simulink library "lib_all"  (Simulink 7.6)
settings: Solver=ode45  SolverMode=SingleTasking  StartTime=0.0  StopTime=10.0  RelTol=1e-3
values from neighbouring files (symbols this model uses):
  map_lib_03 = [6.8679 7.0159 7.4571 8.1849 9.1892 10.456 11.97 13.71 15.655 17.781 20.063 22.474 ... (803 elements, 73x11)]    [scr_simdata.mat]    (also in scr_simdata_all.mat)
  map_lib_06 = [2.5784 2.6727 2.9588 3.4453 4.1444 5.068 6.226 7.6242 9.2634 11.139 13.24 15.552 ... (803 elements, 73x11)]    [scr_simdata.mat]    (also in scr_simdata_all.mat)
  map_lib_09 = [0.0025784 0.0026727 0.0029588 0.0034453 0.0041444 0.005068 0.006226 0.0076242 0.0092634 0.011139 0.01324 0.015552 ... (803 elements, 73x11)]    [scr_simdata.mat]    (also in scr_simdata_all.mat)
  map_lib_10 = [2.74 2.75 2.76 2.77 2.78 2.79 2.8 2.81 2.82 2.83 2.84 2.85 ... (81 elements, 1x81)]    [scr_simdata.mat]    (also in scr_simdata_all.mat)
  map_lib_11 = [1.1983 1.2027 1.207 1.2113 1.2156 1.2198 1.2241 1.2283 1.2326 1.2368 1.241 1.2451 ... (81 elements, 1x81)]    [scr_simdata.mat]    (also in scr_simdata_all.mat)
  map_lib_12 = [2.74 2.75 2.76 2.77 2.78 2.79 2.8 2.81 2.82 2.83 2.84 2.85 ... (81 elements, 1x81)]    [scr_simdata.mat]    (also in scr_simdata_all.mat)
  map_lib_13 = [1.74 1.75 1.76 1.77 1.78 1.79 1.8 1.81 1.82 1.83 1.84 1.85 ... (81 elements, 1x81)]    [scr_simdata.mat]    (also in scr_simdata_all.mat)
  map_lib_16 = [0.00048828125 1.6159 1.8962 2.0821 2.2251 2.3426 2.4433 2.5318 2.611 2.683 2.749 2.8101 ... (1419 elements, 129x11)]    [scr_simdata.mat]    (also in scr_simdata_all.mat)
  map_mr2_01 = [0 800 1600 2400 3200 4000]    [scr_simdata.mat]    (also in scr_simdata_all.mat)
  map_mr2_02 = [1.32 1.32 1.33 1.34 1.36 1.38]    [scr_simdata.mat]    (also in scr_simdata_all.mat)
  map_mr2_03 = [0 800 1600 2400 3200 4000]    [scr_simdata.mat]    (also in scr_simdata_all.mat)
  map_mr2_04 = [1.32 1.32 1.33 1.34 1.36 1.38]    [scr_simdata.mat]    (also in scr_simdata_all.mat)
  map_mr2_05 = [0 800 1600 2400 3200 4000]    [scr_simdata.mat]    (also in scr_simdata_all.mat)
  map_mr2_06 = [1.32 1.32 1.33 1.34 1.36 1.38]    [scr_simdata.mat]    (also in scr_simdata_all.mat)
  Parameter1 = 6    [scr_simdata.mat]    (also in scr_simdata_all.mat)
  Parameter101 = 30    [scr_simdata.mat]    (also in scr_simdata_all.mat)
  Parameter102 = 12    [scr_simdata.mat]    (also in scr_simdata_all.mat)
  Parameter103 = -240    [scr_simdata.mat]    (also in scr_simdata_all.mat)
  Parameter108 = 48    [scr_simdata.mat]    (also in scr_simdata_all.mat)
  Parameter120 = 16    [scr_simdata.mat]    (also in scr_simdata_all.mat)
  Parameter121 = 87    [scr_simdata.mat]    (also in scr_simdata_all.mat)
  Parameter123 = 78    [scr_simdata.mat]    (also in scr_simdata_all.mat)
  Parameter124 = 60    [scr_simdata.mat]    (also in scr_simdata_all.mat)
  Parameter125 = -480    [scr_simdata.mat]    (also in scr_simdata_all.mat)
  Parameter130 = 96    [scr_simdata.mat]    (also in scr_simdata_all.mat)
  Parameter131 = 3    [scr_simdata.mat]    (also in scr_simdata_all.mat)
  Parameter144 = 3    [scr_simdata.mat]    (also in scr_simdata_all.mat)
  Parameter18 = 128    [scr_simdata.mat]    (also in scr_simdata_all.mat)
  Parameter19 = 16    [scr_simdata.mat]    (also in scr_simdata_all.mat)
  Parameter2 = 12    [scr_simdata.mat]    (also in scr_simdata_all.mat)
  Parameter20 = 7    [scr_simdata.mat]    (also in scr_simdata_all.mat)
  Parameter21 = 4    [scr_simdata.mat]    (also in scr_simdata_all.mat)
  Parameter3 = 18    [scr_simdata.mat]    (also in scr_simdata_all.mat)
  Parameter32 = 1    [scr_simdata.mat]    (also in scr_simdata_all.mat)
  Parameter34 = 24    [scr_simdata.mat]    (also in scr_simdata_all.mat)
  Parameter4 = 138    [scr_simdata.mat]    (also in scr_simdata_all.mat)
  Parameter42 = 48    [scr_simdata.mat]    (also in scr_simdata_all.mat)
  Parameter5 = 135    [scr_simdata.mat]    (also in scr_simdata_all.mat)
  Parameter55 = 0    [scr_simdata.mat]    (also in scr_simdata_all.mat)
  Parameter56 = 0    [scr_simdata.mat]    (also in scr_simdata_all.mat)
  Parameter57 = 1    [scr_simdata.mat]    (also in scr_simdata_all.mat)
  Parameter58 = 1    [scr_simdata.mat]    (also in scr_simdata_all.mat)
  Parameter59 = 2    [scr_simdata.mat]    (also in scr_simdata_all.mat)
  Parameter6 = 132    [scr_simdata.mat]    (also in scr_simdata_all.mat)
  Parameter60 = 2    [scr_simdata.mat]    (also in scr_simdata_all.mat)
  Parameter63 = 144    [scr_simdata.mat]    (also in scr_simdata_all.mat)
  Parameter7 = 126    [scr_simdata.mat]    (also in scr_simdata_all.mat)
  Parameter76 = 64    [scr_simdata.mat]    (also in scr_simdata_all.mat)
  Parameter77 = 135    [scr_simdata.mat]    (also in scr_simdata_all.mat)
  Parameter79 = 126    [scr_simdata.mat]    (also in scr_simdata_all.mat)
  Parameter80 = 108    [scr_simdata.mat]    (also in scr_simdata_all.mat)
  Parameter81 = 0    [scr_simdata.mat]    (also in scr_simdata_all.mat)
  Parameter86 = 0    [scr_simdata.mat]    (also in scr_simdata_all.mat)
  Parameter98 = 112    [scr_simdata.mat]    (also in scr_simdata_all.mat)
  Parameter99 = 39    [scr_simdata.mat]    (also in scr_simdata_all.mat)
undefined symbols (not defined in the file or in its folder): cylvolkp_hih_map2h, cylvolkp_map2h, pk1k_map2h
libraries: simulink x2
(part 7 of 36)

== system lib_all/SubSystem1593/SubSystem1595/SubSystem1597 ==
  note: ***編集禁止***
  In1 : Inport
  In2 : Inport  Port=2
  LO1 : Logic
  RO1 : RelationalOperator  Operator=<  InputSameDT=on
  RO2 : RelationalOperator  Operator=~=  InputSameDT=on
  single1 : DataTypeConversion  OutDataTypeStr=single  SaturateOnIntegerOverflow=on  RndMeth=Zero
  single2 : DataTypeConversion  OutDataTypeStr=single  SaturateOnIntegerOverflow=on  RndMeth=Zero
  Out1 : Outport
  wires:
  In2/1 -> single2/1
  In1/1 -> single1/1
  single1/1 -> RO1/1, RO2/1
  single2/1 -> RO1/2, RO2/2
  RO1/1 -> LO1/1
  RO2/1 -> LO1/2
  LO1/1 -> Out1/1

== system lib_all/SubSystem1593/SubSystem1595/SubSystem1598 ==
  note: Text303
  note: Text302
  Block4283 : Inport
  Block4284 : Inport  Port=2
  Block4285 : Inport  Port=3
  Block4286 : Constant  Value=720  OutDataTypeStr=double  SampleTime=-1
  Block4287 : Constant  Value=0  OutDataTypeStr=double  SampleTime=-1
  Block4288 : Constant  Value=0  OutDataTypeStr=double  SampleTime=-1
  Block4289 : Constant  Value=5  OutDataTypeStr=double  SampleTime=-1
  Block4290 : DataStoreRead  DataStoreName=DSM50
  Block4291 : DataStoreRead  DataStoreName=DSM35
  Block4292 : Gain  Gain=5  SaturateOnIntegerOverflow=on  OutDataTypeStr=Inherit: Same as input  ParamDataTypeStr=Inherit: Same as input
      settings: AttributesFormatString=Attribute841
  Block4293 : Gain  Gain=5  SaturateOnIntegerOverflow=on  OutDataTypeStr=Inherit: Same as input  ParamDataTypeStr=Inherit: Same as input
      settings: AttributesFormatString=Attribute842
  Block4294 : Gain  Gain=1/5  SaturateOnIntegerOverflow=on  OutDataTypeStr=Inherit: Same as input  ParamDataTypeStr=Inherit: Same as input
      settings: AttributesFormatString=Attribute843
  Block4295 : Product  Inputs=*/  OutDataTypeStr=double  SaturateOnIntegerOverflow=on  RndMeth=Zero  InputSameDT=on
  Block4296 : Rounding
  Block4297 : Rounding
  Block4298 : Sum  Inputs=+-+-  OutDataTypeStr=double  RndMeth=Zero  SaturateOnIntegerOverflow=on  InputSameDT=on
  Block4299 : Sum  Inputs=-+  OutDataTypeStr=double  RndMeth=Zero  SaturateOnIntegerOverflow=on  InputSameDT=on
  SubSystem1599 : Subsystem  TreatAsAtomicUnit=on  mask(***ATC.DIVISION***){bd_version=1.01.A, r0=チェック有り, r1=, r2=, r3=, r4=, r5=, r6=, r7=, r8=, r9=, c0=, c1=, c2=, c3=, c4=, c5=, c6=, c7=, c8=, c9=}
      mask prompts: bd_version "Version"
      mask help: これはAutoCからのブロックです．編集禁止．
      settings: OpenFcn=warndlg('変更禁止!');
      mask Help: web(ui_blockhelpmng(gcb));
  SubSystem1600 : Subsystem  TreatAsAtomicUnit=on  mask(***ATC.DSRVEC***){bd_version=1.01.A, r0=DSM95, r1=-1, r2=, r3=, r4=, r5=, r6=, r7=, r8=, r9=, c0=, c1=, c2=, c3=, c4=, c5=, c6=, c7=, c8=, c9=}
      mask prompts: bd_version "Version"
      mask help: これはAutoCからのブロックです．編集禁止．
      settings: OpenFcn=warndlg('変更禁止!');
      mask Help: web(ui_blockhelpmng(gcb));
      mask Display: disp('ATC.DSRVEC')
  SubSystem1601 : Subsystem
      settings: RTWSystemCode=Inline
  Block4308 : Outport
  Block4309 : Outport  Port=2
  wires:
  Block4297/1 -> Block4308/1
  Block4294/1 -> Block4297/1
  Block4293/1 -> Block4298/1
  Block4290/1 -> Block4298/2
  SubSystem1600/1(Output) -> SubSystem1599/1(mult)
  Block4287/1 -> SubSystem1599/3(init)
  SubSystem1599/1(Out) -> Block4298/3  "SigLabel147"
  Block4283/1 -> Block4293/1
  SubSystem1601/1(Block4307) -> Block4294/1, Block4299/2, Block4295/1
  Block4285/1 -> Block4298/4
  Block4286/1 -> SubSystem1601/1(Block4300)
  Block4298/1 -> SubSystem1601/2(Block4301)  "SigLabel146"
  Block4288/1 -> SubSystem1601/3(Block4302)
  Block4284/1 -> SubSystem1600/1(element)
  Block4289/1 -> Block4295/2
  Block4295/1 -> Block4296/1
  Block4296/1 -> Block4292/1
  Block4292/1 -> Block4299/1
  Block4299/1 -> Block4309/1
  Block4291/1 -> SubSystem1599/2(div)

== system lib_all/SubSystem1593/SubSystem1595/SubSystem1604 ==
  note: Text306
  note: Text305
  note: Text304
  Block4310 : Inport
  Block4311 : Inport  Port=2
  Block4312 : Inport  Port=3
  Block4313 : Constant  Value=0  OutDataTypeStr=double  SampleTime=-1
  Block4314 : Constant  OutDataTypeStr=double  SampleTime=-1
  Block4315 : Constant  OutDataTypeStr=double  SampleTime=-1
  Block4316 : Constant  Value=0  OutDataTypeStr=double  SampleTime=-1
  Block4317 : Constant  Value=2  OutDataTypeStr=double  SampleTime=-1
  Block4318 : Constant  OutDataTypeStr=double  SampleTime=-1
  Block4319 : Constant  Value=143  OutDataTypeStr=double  SampleTime=-1
  Block4320 : Demux  Outputs=2
  Block4321 : Logic  Operator=NOT
  Block4322 : Mux  Inputs=2
  Block4323 : Mux  Inputs=2
  Block4324 : Sum  OutDataTypeStr=double  RndMeth=Zero  SaturateOnIntegerOverflow=on  InputSameDT=on
  Block4325 : Sum  OutDataTypeStr=double  RndMeth=Zero  SaturateOnIntegerOverflow=on  InputSameDT=on
  Block{4326..4329} (4 alike) : Switch  Threshold=0.5  RndMeth=Zero  SaturateOnIntegerOverflow=on  InputSameDT=on
  SubSystem1605 : Subsystem
  SubSystem1606 : Subsystem
  SubSystem1607 : Subsystem
  SubSystem1608 : Subsystem
  SubSystem1609 : Subsystem  TreatAsAtomicUnit=on
  SubSystem1618 : Subsystem  TreatAsAtomicUnit=on
  SubSystem1628 : Subsystem  TreatAsAtomicUnit=on
  SubSystem1638 : Subsystem  TreatAsAtomicUnit=on
  Block4389 : Outport
  Block4390 : Outport  Port=2
  wires:
  Block4328/1 -> Block4325/2
  Block4318/1 -> Block4325/1
  Block4310/1 -> SubSystem1605/1(In1), Block4324/2, Block4322/1
  Block4319/1 -> SubSystem1605/2(In2), Block4323/1
  Block4311/1 -> SubSystem1606/1(In1), SubSystem1607/1(In1), SubSystem1608/1(In1)
  Block4313/1 -> SubSystem1606/2(In2)
  SubSystem1606/1(Out1) -> Block4326/2, SubSystem1609/Enable
  SubSystem1609/1(Block4343) -> Block4326/1
  Block4314/1 -> SubSystem1607/2(In2)
  SubSystem1607/1(Out1) -> Block4327/2, SubSystem1618/Enable
  SubSystem1618/1(Block4358) -> Block4327/1
  Block4327/1 -> Block4326/3
  Block4325/1 -> SubSystem1609/1(Block4330), SubSystem1618/1(Block4344), SubSystem1628/1(Block4359), SubSystem1638/1(Block4374)
  Block4320/1 -> Block4389/1
  Block4320/2 -> Block4390/1
  Block4326/1 -> Block4320/1
  SubSystem1605/1(Out1) -> Block4328/2
  Block4315/1 -> Block4324/1
  Block4316/1 -> Block4323/2
  Block4323/1 -> Block4328/3
  Block4324/1 -> Block4322/2
  Block4322/1 -> Block4328/1
  Block4317/1 -> SubSystem1608/2(In2)
  SubSystem1608/1(Out1) -> Block4321/1, SubSystem1628/Enable, Block4329/2
  Block4321/1 -> SubSystem1638/Enable
  SubSystem1628/1(Block4373) -> Block4329/1
  SubSystem1638/1(Block4388) -> Block4329/3
  Block4329/1 -> Block4327/3
  Block4312/1 -> SubSystem1609/2(Block4331), SubSystem1618/2(Block4345), SubSystem1628/2(Block4360), SubSystem1638/2(Block4375)

== system lib_all/SubSystem1593/SubSystem1648/SubSystem1650 ==
  note: Text308
  Block4396 : Inport
  Block4397 : Inport  Port=2
  Block4398 : Inport  Port=3
  Block4399 : Constant  Value=0  OutDataTypeStr=double  SampleTime=-1
  Block4400 : Constant  Value=720  OutDataTypeStr=double  SampleTime=-1
  Block4401 : Gain  Gain=5  SaturateOnIntegerOverflow=on  OutDataTypeStr=Inherit: Same as input  ParamDataTypeStr=Inherit: Same as input
      settings: AttributesFormatString=Attribute854
  Block4402 : Sum  Inputs=+-  OutDataTypeStr=double  RndMeth=Zero  SaturateOnIntegerOverflow=on  InputSameDT=on
  SubSystem1651 : Subsystem
      settings: RTWFcnNameOpts=Use subsystem name
  SubSystem1656 : Subsystem
      settings: RTWSystemCode=Inline
  Block4428 : Outport
  wires:
  Block4401/1 -> Block4402/1
  SubSystem1651/1(Block4421) -> Block4401/1
  Block4396/1 -> SubSystem1651/1(Block4403)
  Block4397/1 -> SubSystem1651/2(Block4404)
  Block4398/1 -> Block4402/2
  Block4402/1 -> SubSystem1656/2(Block4423)
  Block4400/1 -> SubSystem1656/1(Block4422)
  Block4399/1 -> SubSystem1656/3(Block4424)
  SubSystem1656/1(Block4427) -> Block4428/1

== system lib_all/SubSystem1593/SubSystem1648/SubSystem1658 ==
  note: Text309
  Block4429 : Inport
  Block4430 : Inport  Port=2
  Block4431 : Constant  Value=360  OutDataTypeStr=double  SampleTime=-1
  Block4432 : Constant  Value=360  OutDataTypeStr=double  SampleTime=-1
  Block4433 : Sum  Inputs=+-  OutDataTypeStr=double  RndMeth=Zero  SaturateOnIntegerOverflow=on  InputSameDT=on
  Block4434 : Switch  Threshold=0.5  RndMeth=Zero  SaturateOnIntegerOverflow=on  InputSameDT=on
  Block4435 : Lookup2D  RowIndex=cylvolkp_map2h_y  ColumnIndex=cylvolkp_map2h_x  Table=cylvolkp_map2h  SaturateOnIntegerOverflow=on  InputSameDT=on
      settings: AttributesFormatString=<%<OutputValues>>\nAttribute863
  SubSystem1659 : Subsystem
  Block4436 : Outport
  wires:
  Block4430/1 -> SubSystem1659/1(In1), Block4434/3, Block4433/1
  Block4432/1 -> SubSystem1659/2(In2)
  SubSystem1659/1(Out1) -> Block4434/2
  Block4433/1 -> Block4434/1
  Block4431/1 -> Block4433/2
  Block4435/1 -> Block4436/1
  Block4429/1 -> Block4435/2
  Block4434/1 -> Block4435/1  "SigLabel149"

== system lib_all/SubSystem1662/SubSystem1664/SubSystem1665 ==
  Block4465 : Inport
  Block4466 : Inport  Port=2
  Block4467 : Constant  Value=Parameter32  OutDataTypeStr=double  SampleTime=-1
  Block4468 : Constant  Value=2  OutDataTypeStr=double  SampleTime=-1
  Block4469 : Constant  Value=Parameter42  OutDataTypeStr=double  SampleTime=-1
  Block4470 : Constant  Value=0  OutDataTypeStr=double  SampleTime=-1
  Block4471 : Constant  Value=72  OutDataTypeStr=double  SampleTime=-1
  Block4472 : Constant  Value=Parameter34  OutDataTypeStr=double  SampleTime=-1
  Block4473 : Logic  Operator=OR
  Block4474 : Sum  Inputs=+-  OutDataTypeStr=double  RndMeth=Zero  SaturateOnIntegerOverflow=on  InputSameDT=on
  Block4475 : Switch  Threshold=0.5  RndMeth=Zero  SaturateOnIntegerOverflow=on  InputSameDT=on
  Block4476 : Switch  Threshold=0.5  RndMeth=Zero  SaturateOnIntegerOverflow=on  InputSameDT=on
  SubSystem1666 : Subsystem
  SubSystem1667 : Subsystem
  SubSystem1668 : Subsystem
      settings: RTWSystemCode=Inline
  Block4483 : Outport
  wires:
  Block4475/1 -> Block4474/2
  Block4472/1 -> Block4475/3
  Block4469/1 -> Block4475/1
  Block4473/1 -> Block4476/2
  SubSystem1667/1(Out1) -> Block4473/2
  SubSystem1666/1(Out1) -> Block4475/2, Block4473/1
  Block4468/1 -> SubSystem1667/2(In2)
  Block4467/1 -> SubSystem1666/1(In1)
  Block4466/1 -> SubSystem1667/1(In1), SubSystem1666/2(In2)
  Block4465/1 -> Block4474/1, Block4476/3
  Block4474/1 -> SubSystem1668/2(Block4478)
  Block4470/1 -> SubSystem1668/3(Block4479)
  Block4471/1 -> SubSystem1668/1(Block4477)
  SubSystem1668/1(Block4482) -> Block4476/1
  Block4476/1 -> Block4483/1

== system lib_all/SubSystem1662/SubSystem1664/SubSystem1670 ==
  Block4484 : Inport
  Block4485 : Inport  Port=2
  Block4486 : Inport  Port=3
  Block4487 : Sum  OutDataTypeStr=double  RndMeth=Zero  SaturateOnIntegerOverflow=on  InputSameDT=on
  Block4488 : Switch  Threshold=0.5  RndMeth=Zero  SaturateOnIntegerOverflow=on  InputSameDT=on
  SubSystem1671 : Subsystem
  Block4489 : Outport
  wires:
  Block4484/1 -> Block4487/1
  Block4488/1 -> Block4489/1
  Block4487/1 -> Block4488/1
  SubSystem1671/1(Out1) -> Block4488/2
  Block4486/1 -> SubSystem1671/2(In2)
  Block4485/1 -> Block4487/2, Block4488/3, SubSystem1671/1(In1)

== system lib_all/SubSystem1662/SubSystem1672/SubSystem1673 ==
  note: ***編集禁止***
  In1 : Inport
  In2 : Inport  Port=2
  RO1 : RelationalOperator  Operator=>  InputSameDT=on
  single1 : DataTypeConversion  OutDataTypeStr=single  SaturateOnIntegerOverflow=on  RndMeth=Zero
  single2 : DataTypeConversion  OutDataTypeStr=single  SaturateOnIntegerOverflow=on  RndMeth=Zero
  Out1 : Outport
  wires:
  RO1/1 -> Out1/1
  In2/1 -> single2/1
  In1/1 -> single1/1
  single1/1 -> RO1/1
  single2/1 -> RO1/2

== system lib_all/SubSystem1674/SubSystem1687/SubSystem1689 ==
  note: ***編集禁止***
  In1 : Inport
  In2 : Inport  Port=2
  RO1 : RelationalOperator  Operator===  InputSameDT=on
  single1 : DataTypeConversion  OutDataTypeStr=single  SaturateOnIntegerOverflow=on  RndMeth=Zero
  single2 : DataTypeConversion  OutDataTypeStr=single  SaturateOnIntegerOverflow=on  RndMeth=Zero
  Out1 : Outport
  wires:
  single2/1 -> RO1/2
  single1/1 -> RO1/1
  In1/1 -> single1/1
  In2/1 -> single2/1
  RO1/1 -> Out1/1

== system lib_all/SubSystem1674/SubSystem1687/SubSystem1690 ==
  note: Text320
  Block4577 : Inport
  Block4578 : Inport  Port=2
  Block4579 : Constant  Value=0  OutDataTypeStr=double  SampleTime=-1
  Block4580 : Constant  Value=720  OutDataTypeStr=double  SampleTime=-1
  Block4581 : Gain  Gain=5  SaturateOnIntegerOverflow=on  OutDataTypeStr=Inherit: Same as input  ParamDataTypeStr=Inherit: Same as input
      settings: AttributesFormatString=Attribute891
  SubSystem1691 : Subsystem
      settings: RTWFcnNameOpts=Use subsystem name
  SubSystem1696 : Subsystem
      settings: RTWSystemCode=Inline
  Block4607 : Outport
  wires:
  Block4579/1 -> SubSystem1696/3(Block4603)
  Block4580/1 -> SubSystem1696/1(Block4601)
  SubSystem1696/1(Block4606) -> Block4607/1
  Block4577/1 -> SubSystem1691/1(Block4582)
  Block4578/1 -> SubSystem1691/2(Block4583)
  SubSystem1691/1(Block4600) -> Block4581/1
  Block4581/1 -> SubSystem1696/2(Block4602)

== system lib_all/SubSystem1674/SubSystem1687/SubSystem1698 ==
  Block4608 : Inport
  Block4609 : Inport  Port=2
  Block4610 : Inport  Port=3
  Block4611 : EnablePort
  Block4612 : DataStoreWrite  DataStoreName=DSM12
  Block4613 : DataStoreWrite  DataStoreName=DSM14
  Block4614 : DataStoreWrite  DataStoreName=DSM13
  wires:
  Block4609/1 -> Block4613/1
  Block4610/1 -> Block4614/1
  Block4608/1 -> Block4612/1

== system lib_all/SubSystem1674/SubSystem1687/SubSystem1699 ==
  note: Text321
  Block4615 : Inport
  Block4616 : Inport  Port=2
  Block4617 : Constant  Value=360  OutDataTypeStr=double  SampleTime=-1
  Block4618 : Constant  Value=360  OutDataTypeStr=double  SampleTime=-1
  Block4619 : Sum  Inputs=+-  OutDataTypeStr=double  RndMeth=Zero  SaturateOnIntegerOverflow=on  InputSameDT=on
  Block4620 : Switch  Threshold=0.5  RndMeth=Zero  SaturateOnIntegerOverflow=on  InputSameDT=on
  Block4621 : Lookup2D  RowIndex=cylvolkp_hih_map2h_y  ColumnIndex=cylvolkp_hih_map2h_x  Table=cylvolkp_hih_map2h  SaturateOnIntegerOverflow=on  InputSameDT=on
      settings: AttributesFormatString=<%<OutputValues>>\nAttribute900
  SubSystem1700 : Subsystem
  Block4622 : Outport
  wires:
  Block4621/1 -> Block4622/1
  Block4617/1 -> Block4619/2
  Block4619/1 -> Block4620/1
  SubSystem1700/1(Out1) -> Block4620/2
  Block4618/1 -> SubSystem1700/2(In2)
  Block4616/1 -> Block4619/1, Block4620/3, SubSystem1700/1(In1)
  Block4615/1 -> Block4621/2
  Block4620/1 -> Block4621/1  "SigLabel153"

== system lib_all/SubSystem1674/SubSystem1701/SubSystem1703 ==
  note: Text325
  note: Text324
  Block4633 : Inport
  Block4634 : Inport  Port=2
  Block4635 : Constant  Value=0  OutDataTypeStr=double  SampleTime=-1
  Block4636 : Constant  Value=0  OutDataTypeStr=double  SampleTime=-1
  Block4637 : Constant  Value=720  OutDataTypeStr=double  SampleTime=-1
  Block4638 : Constant  Value=5  OutDataTypeStr=double  SampleTime=-1
  Block4639 : DataStoreRead  DataStoreName=DSM50
  Block4640 : DataStoreRead  DataStoreName=DSM35
  Block4641 : Gain  Gain=5  SaturateOnIntegerOverflow=on  OutDataTypeStr=Inherit: Same as input  ParamDataTypeStr=Inherit: Same as input
      settings: AttributesFormatString=Attribute908
  Block4642 : Gain  Gain=5  SaturateOnIntegerOverflow=on  OutDataTypeStr=Inherit: Same as input  ParamDataTypeStr=Inherit: Same as input
      settings: AttributesFormatString=Attribute909
  Block4643 : Gain  Gain=1/5  SaturateOnIntegerOverflow=on  OutDataTypeStr=Inherit: Same as input  ParamDataTypeStr=Inherit: Same as input
      settings: AttributesFormatString=Attribute910
  Block4644 : Product  Inputs=*/  OutDataTypeStr=double  SaturateOnIntegerOverflow=on  RndMeth=Zero  InputSameDT=on
  Block4645 : Rounding
  Block4646 : Rounding
  Block4647 : Sum  Inputs=+-+  OutDataTypeStr=double  RndMeth=Zero  SaturateOnIntegerOverflow=on  InputSameDT=on
  Block4648 : Sum  Inputs=-+  OutDataTypeStr=double  RndMeth=Zero  SaturateOnIntegerOverflow=on  InputSameDT=on
  SubSystem1704 : Subsystem  TreatAsAtomicUnit=on  mask(***ATC.DIVISION***){bd_version=1.01.A, r0=チェック有り, r1=, r2=, r3=, r4=, r5=, r6=, r7=, r8=, r9=, c0=, c1=, c2=, c3=, c4=, c5=, c6=, c7=, c8=, c9=}
      mask prompts: bd_version "Version"
      mask help: これはAutoCからのブロックです．編集禁止．
      settings: OpenFcn=warndlg('変更禁止!');
      mask Help: web(ui_blockhelpmng(gcb));
  SubSystem1705 : Subsystem  TreatAsAtomicUnit=on  mask(***ATC.DSRVEC***){bd_version=1.01.A, r0=DSM95, r1=-1, r2=, r3=, r4=, r5=, r6=, r7=, r8=, r9=, c0=, c1=, c2=, c3=, c4=, c5=, c6=, c7=, c8=, c9=}
      mask prompts: bd_version "Version"
      mask help: これはAutoCからのブロックです．編集禁止．
      settings: OpenFcn=warndlg('変更禁止!');
      mask Help: web(ui_blockhelpmng(gcb));
      mask Display: disp('ATC.DSRVEC')
  SubSystem1706 : Subsystem
      settings: RTWSystemCode=Inline
  Block4657 : Outport
  Block4658 : Outport  Port=2
  wires:
  SubSystem1704/1(Out) -> Block4647/1  "SigLabel155"
  Block4633/1 -> Block4642/1
  Block4641/1 -> Block4648/1
  Block4645/1 -> Block4641/1
  Block4644/1 -> Block4645/1
  SubSystem1706/1(Block4656) -> Block4648/2, Block4643/1, Block4644/1
  Block4636/1 -> SubSystem1706/3(Block4651)
  Block4647/1 -> SubSystem1706/2(Block4650)  "SigLabel154"
  Block4637/1 -> SubSystem1706/1(Block4649)
  Block4634/1 -> SubSystem1705/1(element)
  Block4648/1 -> Block4658/1
  Block4638/1 -> Block4644/2
  Block4635/1 -> SubSystem1704/3(init)
  SubSystem1705/1(Output) -> SubSystem1704/1(mult)
  Block4640/1 -> SubSystem1704/2(div)
  Block4639/1 -> Block4647/2
  Block4642/1 -> Block4647/3
  Block4643/1 -> Block4646/1
  Block4646/1 -> Block4657/1

== system lib_all/SubSystem1674/SubSystem1701/SubSystem1709 ==
  note: Text328
  note: Text327
  note: Text326
  Block4659 : Inport
  Block4660 : Inport  Port=2
  Block4661 : Inport  Port=3
  Block4662 : Constant  Value=0  OutDataTypeStr=double  SampleTime=-1
  Block4663 : Constant  OutDataTypeStr=double  SampleTime=-1
  Block4664 : Constant  OutDataTypeStr=double  SampleTime=-1
  Block4665 : Constant  Value=0  OutDataTypeStr=double  SampleTime=-1
  Block4666 : Constant  OutDataTypeStr=double  SampleTime=-1
  Block4667 : Constant  Value=143  OutDataTypeStr=double  SampleTime=-1
  Block4668 : Demux  Outputs=2
  Block4669 : Logic  Operator=NOT
  Block4670 : Mux  Inputs=2
  Block4671 : Mux  Inputs=2
  Block4672 : Sum  OutDataTypeStr=double  RndMeth=Zero  SaturateOnIntegerOverflow=on  InputSameDT=on
  Block4673 : Sum  OutDataTypeStr=double  RndMeth=Zero  SaturateOnIntegerOverflow=on  InputSameDT=on
  Block4674 : Switch  Threshold=0.5  RndMeth=Zero  SaturateOnIntegerOverflow=on  InputSameDT=on
  Block4675 : Switch  Threshold=0.5  RndMeth=Zero  SaturateOnIntegerOverflow=on  InputSameDT=on
  Block4676 : Switch  Threshold=0.5  RndMeth=Zero  SaturateOnIntegerOverflow=on  InputSameDT=on
  SubSystem1710 : Subsystem
  SubSystem1711 : Subsystem
  SubSystem1712 : Subsystem
  SubSystem1713 : Subsystem  TreatAsAtomicUnit=on
  SubSystem1722 : Subsystem  TreatAsAtomicUnit=on
  SubSystem1732 : Subsystem  TreatAsAtomicUnit=on
  Block4721 : Outport
  Block4722 : Outport  Port=2
  wires:
  Block4673/1 -> SubSystem1732/1(Block4706), SubSystem1722/1(Block4691), SubSystem1713/1(Block4677)
  Block4666/1 -> Block4673/1
  Block4676/1 -> Block4673/2
  Block4661/1 -> SubSystem1732/2(Block4707), SubSystem1722/2(Block4692), SubSystem1713/2(Block4678)
  Block4670/1 -> Block4676/1
  Block4672/1 -> Block4670/2
  Block4671/1 -> Block4676/3
  Block4665/1 -> Block4671/2
  Block4664/1 -> Block4672/1
  SubSystem1710/1(Out1) -> Block4676/2
  Block4674/1 -> Block4668/1
  Block4668/2 -> Block4722/1
  Block4668/1 -> Block4721/1
  SubSystem1732/1(Block4720) -> Block4675/3
  Block4675/1 -> Block4674/3
  Block4669/1 -> SubSystem1732/Enable
  SubSystem1722/1(Block4705) -> Block4675/1
  SubSystem1712/1(Out1) -> Block4669/1, SubSystem1722/Enable, Block4675/2
  Block4663/1 -> SubSystem1712/2(In2)
  SubSystem1713/1(Block4690) -> Block4674/1
  SubSystem1711/1(Out1) -> SubSystem1713/Enable, Block4674/2
  Block4662/1 -> SubSystem1711/2(In2)
  Block4660/1 -> SubSystem1712/1(In1), SubSystem1711/1(In1)
  Block4667/1 -> Block4671/1, SubSystem1710/2(In2)
  Block4659/1 -> Block4670/1, Block4672/2, SubSystem1710/1(In1)

== system lib_all/SubSystem1742/SubSystem1745/SubSystem1746 ==
  Block4742 : Inport
  Block4743 : Inport  Port=2
  Block4744 : Constant  Value=Parameter32  OutDataTypeStr=double  SampleTime=-1
  Block4745 : Constant  Value=2  OutDataTypeStr=double  SampleTime=-1
  Block4746 : Constant  Value=Parameter42  OutDataTypeStr=double  SampleTime=-1
  Block4747 : Constant  Value=0  OutDataTypeStr=double  SampleTime=-1
  Block4748 : Constant  Value=72  OutDataTypeStr=double  SampleTime=-1
  Block4749 : Constant  Value=Parameter34  OutDataTypeStr=double  SampleTime=-1
  Block4750 : Logic  Operator=OR
  Block4751 : Sum  Inputs=+-  OutDataTypeStr=double  RndMeth=Zero  SaturateOnIntegerOverflow=on  InputSameDT=on
  Block4752 : Switch  Threshold=0.5  RndMeth=Zero  SaturateOnIntegerOverflow=on  InputSameDT=on
  Block4753 : Switch  Threshold=0.5  RndMeth=Zero  SaturateOnIntegerOverflow=on  InputSameDT=on
  SubSystem1747 : Subsystem
  SubSystem1748 : Subsystem
  SubSystem1749 : Subsystem
      settings: RTWSystemCode=Inline
  Block4760 : Outport
  wires:
  Block4753/1 -> Block4760/1
  Block4752/1 -> Block4751/2
  Block4749/1 -> Block4752/3
  Block4746/1 -> Block4752/1
  Block4750/1 -> Block4753/2
  SubSystem1748/1(Out1) -> Block4750/2
  SubSystem1747/1(Out1) -> Block4752/2, Block4750/1
  Block4745/1 -> SubSystem1748/2(In2)
  Block4744/1 -> SubSystem1747/1(In1)
  Block4743/1 -> SubSystem1748/1(In1), SubSystem1747/2(In2)
  Block4742/1 -> Block4751/1, Block4753/3
  Block4751/1 -> SubSystem1749/2(Block4755)
  Block4747/1 -> SubSystem1749/3(Block4756)
  Block4748/1 -> SubSystem1749/1(Block4754)
  SubSystem1749/1(Block4759) -> Block4753/1

== system lib_all/SubSystem1742/SubSystem1745/SubSystem1751 ==
  Block4761 : Inport
  Block4762 : Inport  Port=2
  Block4763 : Inport  Port=3
  Block4764 : Sum  OutDataTypeStr=double  RndMeth=Zero  SaturateOnIntegerOverflow=on  InputSameDT=on
  Block4765 : Switch  Threshold=0.5  RndMeth=Zero  SaturateOnIntegerOverflow=on  InputSameDT=on
  SubSystem1752 : Subsystem
  Block4766 : Outport
  wires:
  Block4761/1 -> Block4764/1
  Block4765/1 -> Block4766/1
  Block4764/1 -> Block4765/1
  SubSystem1752/1(Out1) -> Block4765/2
  Block4763/1 -> SubSystem1752/2(In2)
  Block4762/1 -> Block4764/2, Block4765/3, SubSystem1752/1(In1)

== system lib_all/SubSystem1742/SubSystem1754/SubSystem1755 ==
  note: ***編集禁止***
  In1 : Inport
  In2 : Inport  Port=2
  RO1 : RelationalOperator  Operator=>  InputSameDT=on
  single1 : DataTypeConversion  OutDataTypeStr=single  SaturateOnIntegerOverflow=on  RndMeth=Zero
  single2 : DataTypeConversion  OutDataTypeStr=single  SaturateOnIntegerOverflow=on  RndMeth=Zero
  Out1 : Outport
  wires:
  RO1/1 -> Out1/1
  In2/1 -> single2/1
  In1/1 -> single1/1
  single1/1 -> RO1/1
  single2/1 -> RO1/2

== system lib_all/SubSystem1756/SubSystem1759/SubSystem1760 ==
  Block4801 : Inport
  Block4802 : Inport  Port=2
  Block4803 : Constant  Value=Parameter32  OutDataTypeStr=double  SampleTime=-1
  Block4804 : Constant  Value=2  OutDataTypeStr=double  SampleTime=-1
  Block4805 : Constant  Value=Parameter42  OutDataTypeStr=double  SampleTime=-1
  Block4806 : Constant  Value=0  OutDataTypeStr=double  SampleTime=-1
  Block4807 : Constant  Value=72  OutDataTypeStr=double  SampleTime=-1
  Block4808 : Constant  Value=Parameter34  OutDataTypeStr=double  SampleTime=-1
  Block4809 : Logic  Operator=OR
  Block4810 : Sum  Inputs=+-  OutDataTypeStr=double  RndMeth=Zero  SaturateOnIntegerOverflow=on  InputSameDT=on
  Block4811 : Switch  Threshold=0.5  RndMeth=Zero  SaturateOnIntegerOverflow=on  InputSameDT=on
  Block4812 : Switch  Threshold=0.5  RndMeth=Zero  SaturateOnIntegerOverflow=on  InputSameDT=on
  SubSystem1761 : Subsystem
  SubSystem1762 : Subsystem
  SubSystem1763 : Subsystem
      settings: RTWSystemCode=Inline
  Block4819 : Outport
  wires:
  SubSystem1763/1(Block4818) -> Block4812/1
  Block4807/1 -> SubSystem1763/1(Block4813)
  Block4806/1 -> SubSystem1763/3(Block4815)
  Block4810/1 -> SubSystem1763/2(Block4814)
  Block4801/1 -> Block4812/3, Block4810/1
  Block4802/1 -> SubSystem1761/2(In2), SubSystem1762/1(In1)
  Block4803/1 -> SubSystem1761/1(In1)
  Block4804/1 -> SubSystem1762/2(In2)
  SubSystem1761/1(Out1) -> Block4809/1, Block4811/2
  SubSystem1762/1(Out1) -> Block4809/2
  Block4809/1 -> Block4812/2
  Block4805/1 -> Block4811/1
  Block4808/1 -> Block4811/3
  Block4811/1 -> Block4810/2
  Block4812/1 -> Block4819/1

== system lib_all/SubSystem1756/SubSystem1759/SubSystem1765 ==
  Block4820 : Inport
  Block4821 : Inport  Port=2
  Block4822 : Inport  Port=3
  Block4823 : Sum  OutDataTypeStr=double  RndMeth=Zero  SaturateOnIntegerOverflow=on  InputSameDT=on
  Block4824 : Switch  Threshold=0.5  RndMeth=Zero  SaturateOnIntegerOverflow=on  InputSameDT=on
  SubSystem1766 : Subsystem
  Block4825 : Outport
  wires:
  Block4821/1 -> SubSystem1766/1(In1), Block4824/3, Block4823/2
  Block4822/1 -> SubSystem1766/2(In2)
  SubSystem1766/1(Out1) -> Block4824/2
  Block4823/1 -> Block4824/1
  Block4824/1 -> Block4825/1
  Block4820/1 -> Block4823/1

== system lib_all/SubSystem1756/SubSystem1768/SubSystem1769 ==
  note: ***編集禁止***
  In1 : Inport
  In2 : Inport  Port=2
  RO1 : RelationalOperator  Operator=>  InputSameDT=on
  single1 : DataTypeConversion  OutDataTypeStr=single  SaturateOnIntegerOverflow=on  RndMeth=Zero
  single2 : DataTypeConversion  OutDataTypeStr=single  SaturateOnIntegerOverflow=on  RndMeth=Zero
  Out1 : Outport
  wires:
  single2/1 -> RO1/2
  single1/1 -> RO1/1
  In1/1 -> single1/1
  In2/1 -> single2/1
  RO1/1 -> Out1/1

== system lib_all/SubSystem176/SubSystem178/SubSystem179 ==
  note: ***編集禁止***
  In1 : Inport
  In2 : Inport  Port=2
  LO1 : Logic
  RO1 : RelationalOperator  Operator=<  InputSameDT=on
  RO2 : RelationalOperator  Operator=~=  InputSameDT=on
  single1 : DataTypeConversion  OutDataTypeStr=single  SaturateOnIntegerOverflow=on  RndMeth=Zero
  single2 : DataTypeConversion  OutDataTypeStr=single  SaturateOnIntegerOverflow=on  RndMeth=Zero
  Out1 : Outport
  wires:
  In2/1 -> single2/1
  In1/1 -> single1/1
  single1/1 -> RO1/1, RO2/1
  single2/1 -> RO1/2, RO2/2
  RO1/1 -> LO1/1
  RO2/1 -> LO1/2
  LO1/1 -> Out1/1

== system lib_all/SubSystem176/SubSystem178/SubSystem180 ==
  Block390 : Inport
  Block391 : Constant  Value=Parameter102  OutDataTypeStr=double  SampleTime=-1
  Block392 : Constant  Value=Parameter120  OutDataTypeStr=double  SampleTime=-1
  Block393 : Constant  Value=Parameter86  OutDataTypeStr=double  SampleTime=-1
  Block394 : Constant  Value=Parameter80  OutDataTypeStr=double  SampleTime=-1
  Block395 : Constant  Value=Parameter108  OutDataTypeStr=double  SampleTime=-1
  Block396 : Constant  Value=Parameter130  OutDataTypeStr=double  SampleTime=-1
  Block397 : MultiPortSwitch  zeroidx=off  RndMeth=Zero  SaturateOnIntegerOverflow=on  InputSameDT=on
  Block398 : Mux  Inputs=2
  Block399 : Mux  Inputs=2
  Block400 : Mux  Inputs=2
  Block401 : Outport
  wires:
  Block393/1 -> Block398/2
  Block395/1 -> Block399/2
  Block396/1 -> Block400/2
  Block392/1 -> Block400/1
  Block391/1 -> Block399/1
  Block397/1 -> Block401/1
  Block390/1 -> Block397/1
  Block394/1 -> Block398/1
  Block398/1 -> Block397/2
  Block400/1 -> Block397/4
  Block399/1 -> Block397/3

== system lib_all/SubSystem176/SubSystem178/SubSystem181 ==
  note: ***編集禁止***
  element : Inport
  Inside_DSR : DataStoreRead  DataStoreName=DSM378
  Selector : Selector  IndexOptions=Index vector (port)  Indices=[1 3]  OutputSizes=1
  Output : Outport
  wires:
  Selector/1 -> Output/1
  element/1 -> Selector/2
  Inside_DSR/1 -> Selector/1

== system lib_all/SubSystem176/SubSystem178/SubSystem182 ==
  Block402 : Inport
  Block403 : Inport  Port=2
  Block404 : Inport  Port=3
  Block405 : Inport  Port=4
  Block406 : EnablePort
  Block407 : Constant  OutDataTypeStr=double  SampleTime=-1
  Block408 : Constant  Value=0  OutDataTypeStr=double  SampleTime=-1
  Block409 : Constant  OutDataTypeStr=double  SampleTime=-1
  Block410 : Constant  Value=0  OutDataTypeStr=double  SampleTime=-1
  Block411 : Sum  OutDataTypeStr=double  RndMeth=Zero  SaturateOnIntegerOverflow=on  InputSameDT=on
  Block412 : Sum  OutDataTypeStr=double  RndMeth=Zero  SaturateOnIntegerOverflow=on  InputSameDT=on
  SubSystem183 : Subsystem  TreatAsAtomicUnit=on
      settings: AttributesFormatString=Attribute96  RTWFcnNameOpts=Use subsystem name
  SubSystem236 : Subsystem  TreatAsAtomicUnit=on
      settings: AttributesFormatString=Attribute115  RTWFcnNameOpts=Use subsystem name
  SubSystem289 : Subsystem
  SubSystem296 : Subsystem
  wires:
  Block409/1 -> Block412/2
  Block412/1 -> SubSystem296/3(Block673), SubSystem289/3(Block659)
  Block405/1 -> Block412/1
  Block403/1 -> SubSystem183/2(Block414)
  Block404/1 -> SubSystem236/2(Block536)
  Block407/1 -> Block411/2
  Block402/1 -> SubSystem236/1(Block535), SubSystem183/1(Block413), Block411/1
  Block410/1 -> SubSystem183/4(Block416), SubSystem183/3(Block415)
  Block408/1 -> SubSystem236/4(Block538), SubSystem236/3(Block537)
  SubSystem183/1(Block534) -> SubSystem289/1(Block657)
  Block411/1 -> SubSystem289/2(Block658), SubSystem296/2(Block672)
  SubSystem236/1(Block656) -> SubSystem296/1(Block671)

== system lib_all/SubSystem176/SubSystem303/SubSystem304 ==
  note: ***編集禁止***
  In1 : Inport
  In2 : Inport  Port=2
  RO1 : RelationalOperator  Operator===  InputSameDT=on
  single1 : DataTypeConversion  OutDataTypeStr=single  SaturateOnIntegerOverflow=on  RndMeth=Zero
  single2 : DataTypeConversion  OutDataTypeStr=single  SaturateOnIntegerOverflow=on  RndMeth=Zero
  Out1 : Outport
  wires:
  RO1/1 -> Out1/1
  In2/1 -> single2/1
  In1/1 -> single1/1
  single1/1 -> RO1/1
  single2/1 -> RO1/2

== system lib_all/SubSystem176/SubSystem303/SubSystem305 ==
  Block689 : Inport
  Block690 : EnablePort
  Block691 : Constant  Value=Parameter19  OutDataTypeStr=double  SampleTime=-1
  SubSystem306 : Subsystem  TreatAsAtomicUnit=on
      settings: RTWSystemCode=Inline
  SubSystem308 : Subsystem
  SubSystem318 : Subsystem  TreatAsAtomicUnit=on
  wires:
  Block689/1 -> SubSystem318/2(Block716), SubSystem308/3(Block697)
  Block691/1 -> SubSystem306/1(N)
  SubSystem306/1(action) -> SubSystem318/Trigger
  SubSystem306/2(i) -> SubSystem318/1(Block715)
  SubSystem318/1(Block733) -> SubSystem308/1(Block695)
  SubSystem318/2(Block734) -> SubSystem308/2(Block696)

== system lib_all/SubSystem176/SubSystem303/SubSystem328 ==
  note: ***編集禁止***
  element : Inport
  Inside_DSR : DataStoreRead  DataStoreName=DSM378
  Selector : Selector  IndexOptions=Index vector (port)  Indices=[1 3]  OutputSizes=1
  Output : Outport
  wires:
  Selector/1 -> Output/1
  element/1 -> Selector/2
  Inside_DSR/1 -> Selector/1

== system lib_all/SubSystem176/SubSystem329/SubSystem330 ==
  note: ***編集禁止***
  In1 : Inport
  In2 : Inport  Port=2
  LO1 : Logic  Operator=OR
  RO1 : RelationalOperator  InputSameDT=on
  RO2 : RelationalOperator  Operator===  InputSameDT=on
  single1 : DataTypeConversion  OutDataTypeStr=single  SaturateOnIntegerOverflow=on  RndMeth=Zero
  single2 : DataTypeConversion  OutDataTypeStr=single  SaturateOnIntegerOverflow=on  RndMeth=Zero
  Out1 : Outport
  wires:
  In2/1 -> single2/1
  In1/1 -> single1/1
  single1/1 -> RO1/1, RO2/1
  single2/1 -> RO1/2, RO2/2
  RO1/1 -> LO1/1
  RO2/1 -> LO1/2
  LO1/1 -> Out1/1

== system lib_all/SubSystem176/SubSystem329/SubSystem331 ==
  note: ***編集禁止***
  In1 : Inport
  In2 : Inport  Port=2
  RO1 : RelationalOperator  Operator===  InputSameDT=on
  single1 : DataTypeConversion  OutDataTypeStr=single  SaturateOnIntegerOverflow=on  RndMeth=Zero
  single2 : DataTypeConversion  OutDataTypeStr=single  SaturateOnIntegerOverflow=on  RndMeth=Zero
  Out1 : Outport
  wires:
  RO1/1 -> Out1/1
  In2/1 -> single2/1
  In1/1 -> single1/1
  single1/1 -> RO1/1
  single2/1 -> RO1/2

== system lib_all/SubSystem176/SubSystem329/SubSystem332 ==
  Block739 : EnablePort
  Block740 : Constant  Value=Parameter131  OutDataTypeStr=double  SampleTime=-1
  SubSystem333 : Subsystem  TreatAsAtomicUnit=on
      settings: RTWSystemCode=Inline
  SubSystem335 : Subsystem  TreatAsAtomicUnit=on
      settings: RTWSystemCode=Inline
  SubSystem337 : Subsystem  TreatAsAtomicUnit=on
  SubSystem341 : Subsystem  TreatAsAtomicUnit=on
  SubSystem343 : Subsystem  Priority=2
      settings: AttributesFormatString=Attribute159
  wires:
  Block740/1 -> SubSystem333/1(N), SubSystem335/1(N)
  SubSystem333/1(action) -> SubSystem337/Trigger
  SubSystem333/2(i) -> SubSystem337/1(Block747)
  SubSystem335/1(action) -> SubSystem341/Trigger
  SubSystem335/2(i) -> SubSystem341/1(Block751)

== system lib_all/SubSystem176/SubSystem344/SubSystem345 ==
  note: ***編集禁止***
  element : Inport
  Inside_DSR : DataStoreRead  DataStoreName=DSM378
  Selector : Selector  IndexOptions=Index vector (port)  Indices=[1 3]  OutputSizes=1
  Output : Outport
  wires:
  Selector/1 -> Output/1
  element/1 -> Selector/2
  Inside_DSR/1 -> Selector/1

== system lib_all/SubSystem176/SubSystem344/SubSystem346 ==
  note: ***編集禁止***
  input : Inport
  element : Inport  Port=2
  Assignment : Assignment  IndexOptions=Index vector (port)  Indices=1  OutputSizes=[1 1]
  Inside_DSR : DataStoreRead  DataStoreName=DSM378
  Inside_DSW : DataStoreWrite  DataStoreName=DSM378
  wires:
  element/1 -> Assignment/3
  input/1 -> Assignment/2
  Inside_DSR/1 -> Assignment/1
  Assignment/1 -> Inside_DSW/1

== system lib_all/SubSystem1770/SubSystem1771/EnabledSubsystem ==
  Enable : EnablePort
  Function-Call Generator : simulink/Ports & Subsystems/Function-Call Generator  sample_time=-1  numberOfIterations=1
  Out1 : Outport
  wires:
  Function-Call Generator/1 -> Out1/1

== system lib_all/SubSystem1770/SubSystem1772/EnabledSubsystem ==
  Enable : EnablePort
  Function-Call Generator : simulink/Ports & Subsystems/Function-Call Generator  sample_time=-1  numberOfIterations=1
  Out1 : Outport
  wires:
  Function-Call Generator/1 -> Out1/1

== system lib_all/SubSystem1770/SubSystem1774/SubSystem1775 ==
  note: ***編集禁止***
  In1 : Inport
  In2 : Inport  Port=2
  RO1 : RelationalOperator  Operator===  InputSameDT=on
  single1 : DataTypeConversion  OutDataTypeStr=single  SaturateOnIntegerOverflow=on  RndMeth=Zero
  single2 : DataTypeConversion  OutDataTypeStr=single  SaturateOnIntegerOverflow=on  RndMeth=Zero
  Out1 : Outport
  wires:
  RO1/1 -> Out1/1
  In2/1 -> single2/1
  In1/1 -> single1/1
  single1/1 -> RO1/1
  single2/1 -> RO1/2

== system lib_all/SubSystem1770/SubSystem1774/SubSystem1776 ==
  note: ***編集禁止***
  In1 : Inport
  In2 : Inport  Port=2
  RO1 : RelationalOperator  Operator===  InputSameDT=on
  single1 : DataTypeConversion  OutDataTypeStr=single  SaturateOnIntegerOverflow=on  RndMeth=Zero
  single2 : DataTypeConversion  OutDataTypeStr=single  SaturateOnIntegerOverflow=on  RndMeth=Zero
  Out1 : Outport
  wires:
  RO1/1 -> Out1/1
  In2/1 -> single2/1
  In1/1 -> single1/1
  single1/1 -> RO1/1
  single2/1 -> RO1/2

== system lib_all/SubSystem1770/SubSystem1774/SubSystem1777 ==
  note: ***編集禁止***
  In1 : Inport
  In2 : Inport  Port=2
  RO1 : RelationalOperator  Operator===  InputSameDT=on
  single1 : DataTypeConversion  OutDataTypeStr=single  SaturateOnIntegerOverflow=on  RndMeth=Zero
  single2 : DataTypeConversion  OutDataTypeStr=single  SaturateOnIntegerOverflow=on  RndMeth=Zero
  Out1 : Outport
  wires:
  RO1/1 -> Out1/1
  In2/1 -> single2/1
  In1/1 -> single1/1
  single1/1 -> RO1/1
  single2/1 -> RO1/2

== system lib_all/SubSystem1770/SubSystem1774/SubSystem1778 ==
  Block4852 : EnablePort
  Block4853 : Constant  Value=63  OutDataTypeStr=double  SampleTime=-1
  Block4854 : Constant  OutDataTypeStr=double  SampleTime=-1
  Block4855 : Constant  Value=Parameter55  OutDataTypeStr=double  SampleTime=-1
  Block4856 : Sum  Inputs=|++  OutDataTypeStr=double  RndMeth=Zero  SaturateOnIntegerOverflow=on  InputSameDT=on
  SubSystem1779 : Subsystem  TreatAsAtomicUnit=on
      settings: RTWFcnNameOpts=Use subsystem name
  SubSystem1790 : Subsystem  TreatAsAtomicUnit=on  mask(***ATC.DSWVEC***){bd_version=1.01.A, r0=DSM1, r1=-1, r2=, r3=, r4=, r5=, r6=, r7=, r8=, r9=, c0=, c1=, c2=, c3=, c4=, c5=, c6=, c7=, c8=, c9=}
      mask prompts: bd_version "Version"
      mask help: これはAutoCからのブロックです．編集禁止．
      settings: OpenFcn=warndlg('変更禁止!');
      mask Help: web(ui_blockhelpmng(gcb));
      mask Display: disp('ATC.DSRVEC')
  wires:
  Block4854/1 -> Block4856/2
  Block4856/1 -> SubSystem1790/2(element)
  Block4855/1 -> Block4856/1, SubSystem1779/1(Block4857)
  SubSystem1779/1(Block4890) -> SubSystem1790/1(input)
  Block4853/1 -> SubSystem1779/2(Block4858)

== system lib_all/SubSystem1770/SubSystem1774/SubSystem1791 ==
  Block4891 : EnablePort
  Block4892 : Constant  Value=111  OutDataTypeStr=double  SampleTime=-1
  Block4893 : Constant  OutDataTypeStr=double  SampleTime=-1
  Block4894 : Constant  Value=Parameter57  OutDataTypeStr=double  SampleTime=-1
  Block4895 : Sum  Inputs=|++  OutDataTypeStr=double  RndMeth=Zero  SaturateOnIntegerOverflow=on  InputSameDT=on
  SubSystem1792 : Subsystem  TreatAsAtomicUnit=on
      settings: RTWFcnNameOpts=Use subsystem name
  SubSystem1803 : Subsystem  TreatAsAtomicUnit=on  mask(***ATC.DSWVEC***){bd_version=1.01.A, r0=DSM1, r1=-1, r2=, r3=, r4=, r5=, r6=, r7=, r8=, r9=, c0=, c1=, c2=, c3=, c4=, c5=, c6=, c7=, c8=, c9=}
      mask prompts: bd_version "Version"
      mask help: これはAutoCからのブロックです．編集禁止．
      settings: OpenFcn=warndlg('変更禁止!');
      mask Help: web(ui_blockhelpmng(gcb));
      mask Display: disp('ATC.DSRVEC')
  wires:
  Block4895/1 -> SubSystem1803/2(element)
  Block4893/1 -> Block4895/2
  Block4892/1 -> SubSystem1792/2(Block4897)
  SubSystem1792/1(Block4929) -> SubSystem1803/1(input)
  Block4894/1 -> Block4895/1, SubSystem1792/1(Block4896)

== system lib_all/SubSystem1770/SubSystem1774/SubSystem1804 ==
  Block4930 : EnablePort
  Block4931 : Constant  Value=15  OutDataTypeStr=double  SampleTime=-1
  Block4932 : Constant  OutDataTypeStr=double  SampleTime=-1
  Block4933 : Constant  Value=Parameter59  OutDataTypeStr=double  SampleTime=-1
  Block4934 : Sum  Inputs=|++  OutDataTypeStr=double  RndMeth=Zero  SaturateOnIntegerOverflow=on  InputSameDT=on
  SubSystem1805 : Subsystem  TreatAsAtomicUnit=on
      settings: RTWFcnNameOpts=Use subsystem name
  SubSystem1816 : Subsystem  TreatAsAtomicUnit=on  mask(***ATC.DSWVEC***){bd_version=1.01.A, r0=DSM1, r1=-1, r2=, r3=, r4=, r5=, r6=, r7=, r8=, r9=, c0=, c1=, c2=, c3=, c4=, c5=, c6=, c7=, c8=, c9=}
      mask prompts: bd_version "Version"
      mask help: これはAutoCからのブロックです．編集禁止．
      settings: OpenFcn=warndlg('変更禁止!');
      mask Help: web(ui_blockhelpmng(gcb));
      mask Display: disp('ATC.DSRVEC')
  wires:
  Block4934/1 -> SubSystem1816/2(element)
  Block4932/1 -> Block4934/2
  Block4933/1 -> Block4934/1, SubSystem1805/1(Block4935)
  SubSystem1805/1(Block4968) -> SubSystem1816/1(input)
  Block4931/1 -> SubSystem1805/2(Block4936)

== system lib_all/SubSystem1770/SubSystem1817/SubSystem1818 ==
  note: ***編集禁止***
  In1 : Inport
  In2 : Inport  Port=2
  RO1 : RelationalOperator  Operator===  InputSameDT=on
  single1 : DataTypeConversion  OutDataTypeStr=single  SaturateOnIntegerOverflow=on  RndMeth=Zero
  single2 : DataTypeConversion  OutDataTypeStr=single  SaturateOnIntegerOverflow=on  RndMeth=Zero
  Out1 : Outport
  wires:
  RO1/1 -> Out1/1
  In2/1 -> single2/1
  In1/1 -> single1/1
  single1/1 -> RO1/1
  single2/1 -> RO1/2

== system lib_all/SubSystem1770/SubSystem1817/SubSystem1819 ==
  note: ***編集禁止***
  In1 : Inport
  In2 : Inport  Port=2
  RO1 : RelationalOperator  Operator===  InputSameDT=on
  single1 : DataTypeConversion  OutDataTypeStr=single  SaturateOnIntegerOverflow=on  RndMeth=Zero
  single2 : DataTypeConversion  OutDataTypeStr=single  SaturateOnIntegerOverflow=on  RndMeth=Zero
  Out1 : Outport
  wires:
  RO1/1 -> Out1/1
  In2/1 -> single2/1
  In1/1 -> single1/1
  single1/1 -> RO1/1
  single2/1 -> RO1/2

== system lib_all/SubSystem1770/SubSystem1817/SubSystem1820 ==
  note: ***編集禁止***
  In1 : Inport
  In2 : Inport  Port=2
  RO1 : RelationalOperator  Operator===  InputSameDT=on
  single1 : DataTypeConversion  OutDataTypeStr=single  SaturateOnIntegerOverflow=on  RndMeth=Zero
  single2 : DataTypeConversion  OutDataTypeStr=single  SaturateOnIntegerOverflow=on  RndMeth=Zero
  Out1 : Outport
  wires:
  RO1/1 -> Out1/1
  In2/1 -> single2/1
  In1/1 -> single1/1
  single1/1 -> RO1/1
  single2/1 -> RO1/2

== system lib_all/SubSystem1770/SubSystem1817/SubSystem1821 ==
  Block4978 : EnablePort
  Block4979 : Constant  Value=87  OutDataTypeStr=double  SampleTime=-1
  Block4980 : Constant  OutDataTypeStr=double  SampleTime=-1
  Block4981 : Constant  Value=Parameter56  OutDataTypeStr=double  SampleTime=-1
  Block4982 : Sum  Inputs=|++  OutDataTypeStr=double  RndMeth=Zero  SaturateOnIntegerOverflow=on  InputSameDT=on
  SubSystem1822 : Subsystem  TreatAsAtomicUnit=on
      settings: RTWFcnNameOpts=Use subsystem name
  SubSystem1833 : Subsystem  TreatAsAtomicUnit=on  mask(***ATC.DSWVEC***){bd_version=1.01.A, r0=DSM1, r1=-1, r2=, r3=, r4=, r5=, r6=, r7=, r8=, r9=, c0=, c1=, c2=, c3=, c4=, c5=, c6=, c7=, c8=, c9=}
      mask prompts: bd_version "Version"
      mask help: これはAutoCからのブロックです．編集禁止．
      settings: OpenFcn=warndlg('変更禁止!');
      mask Help: web(ui_blockhelpmng(gcb));
      mask Display: disp('ATC.DSRVEC')
  wires:
  Block4982/1 -> SubSystem1833/2(element)
  Block4980/1 -> Block4982/2
  Block4979/1 -> SubSystem1822/2(Block4984)
  SubSystem1822/1(Block5016) -> SubSystem1833/1(input)
  Block4981/1 -> Block4982/1, SubSystem1822/1(Block4983)

== system lib_all/SubSystem1770/SubSystem1817/SubSystem1834 ==
  Block5017 : EnablePort
  Block5018 : Constant  Value=135  OutDataTypeStr=double  SampleTime=-1
  Block5019 : Constant  OutDataTypeStr=double  SampleTime=-1
  Block5020 : Constant  Value=Parameter58  OutDataTypeStr=double  SampleTime=-1
  Block5021 : Sum  Inputs=|++  OutDataTypeStr=double  RndMeth=Zero  SaturateOnIntegerOverflow=on  InputSameDT=on
  SubSystem1835 : Subsystem  TreatAsAtomicUnit=on
      settings: RTWFcnNameOpts=Use subsystem name
  SubSystem1846 : Subsystem  TreatAsAtomicUnit=on  mask(***ATC.DSWVEC***){bd_version=1.01.A, r0=DSM1, r1=-1, r2=, r3=, r4=, r5=, r6=, r7=, r8=, r9=, c0=, c1=, c2=, c3=, c4=, c5=, c6=, c7=, c8=, c9=}
      mask prompts: bd_version "Version"
      mask help: これはAutoCからのブロックです．編集禁止．
      settings: OpenFcn=warndlg('変更禁止!');
      mask Help: web(ui_blockhelpmng(gcb));
      mask Display: disp('ATC.DSRVEC')
  wires:
  Block5021/1 -> SubSystem1846/2(element)
  Block5019/1 -> Block5021/2
  Block5020/1 -> Block5021/1, SubSystem1835/1(Block5022)
  SubSystem1835/1(Block5055) -> SubSystem1846/1(input)
  Block5018/1 -> SubSystem1835/2(Block5023)

== system lib_all/SubSystem1770/SubSystem1817/SubSystem1847 ==
  Block5056 : EnablePort
  Block5057 : Constant  Value=39  OutDataTypeStr=double  SampleTime=-1
  Block5058 : Constant  OutDataTypeStr=double  SampleTime=-1
  Block5059 : Constant  Value=Parameter60  OutDataTypeStr=double  SampleTime=-1
  Block5060 : Sum  Inputs=|++  OutDataTypeStr=double  RndMeth=Zero  SaturateOnIntegerOverflow=on  InputSameDT=on
  SubSystem1848 : Subsystem  TreatAsAtomicUnit=on
      settings: RTWFcnNameOpts=Use subsystem name
  SubSystem1859 : Subsystem  TreatAsAtomicUnit=on  mask(***ATC.DSWVEC***){bd_version=1.01.A, r0=DSM1, r1=-1, r2=, r3=, r4=, r5=, r6=, r7=, r8=, r9=, c0=, c1=, c2=, c3=, c4=, c5=, c6=, c7=, c8=, c9=}
      mask prompts: bd_version "Version"
      mask help: これはAutoCからのブロックです．編集禁止．
      settings: OpenFcn=warndlg('変更禁止!');
      mask Help: web(ui_blockhelpmng(gcb));
      mask Display: disp('ATC.DSRVEC')
  wires:
  Block5060/1 -> SubSystem1859/2(element)
  Block5058/1 -> Block5060/2
  Block5057/1 -> SubSystem1848/2(Block5062)
  SubSystem1848/1(Block5094) -> SubSystem1859/1(input)
  Block5059/1 -> Block5060/1, SubSystem1848/1(Block5061)
